simulink model "TosAeb"  (R2021a)
settings: Solver=FixedStepDiscrete  StartTime=0.0  StopTime=inf  FixedStep=0.01  RelTol=1e-3
undefined symbols (not defined in the file or in its folder): ACCModSts, AccSts1, AEB_ObjectMovingOrientation, AEB_ObjectMovingState, CN_BTCActive, CN_CurrentGearR, CN_CurrentGearR_DHT, CN_PTCActive, CN_VDCActive, CN_VSC_EspOff, CN_VSC_FCW_AEB_Error, CN_VSC_FCW_AEB_NoDispay, CN_VSC_FCW_AEB_PerfmDgdtn, CN_VSC_FCW_AEB_TempUnAvlbl, CN_VSC_VehStandstill, CNaccelAvoidMax, CNaccelAvoidMin, CNbrakePedalPress, CNcomfortStrLeftAccelMax, CNcomfortStrLeftAccelMin, CNcomfortStrRightAccelMax, CNcomfortStrRightAccelMin, CNDrvDoorOpen, CNDrvModeEco, CNDrvModeNorm, CNDrvModeOther, CNDrvModeSport, CNDrvSBRreminder, CNNull, CNobjInvalidID, CNPassDoorOpen, CNPassSBRreminder, CNPEBSAccelNegInf, CNPEBSAccelNull, CNPEBSTimeInf, CNPEBSTimeNull, CNSTOPMAX, CNSTOPNULL, CNsubjWidth, CNSwtOFF, CNSwtON, CNTTCMAX, CNTTCNULL, CNVehConfigAEBCycNone, CNVehConfigAEBNone, CNVehConfigAEBPedNone, CNVehConfigFCWNone, CNVehConfigJANone, CollisionSeverity, FALSE, FCWSnvty, JACycObjCalcInfoDefaultParams, JAPedObjCalcInfoDefaultParams, JAVehObjCalcInfoDefaultParams, latCycObjCalcInfoDefaultParams, latPedObjCalcInfoDefaultParams, longObjCalcInfoDefaultParams, ModSts, movingStatus, ObjectType ... (+1112)
libraries: simulink x3031, AEBSFunctionLib x50, Ta_Library x33, AEBSStateMachineLib x23, Mylibrary x12, simulink_extras x7, ObjSelect_lib x3, dspstat3 x2
(part 70 of 102)

== system TosAeb/FcwAebJa_Step_sys/ALG_AEBSystem/ALG_AEBController/AEB_LatObjInfoCalcApp/LatCycObjCalc/LatCycObjCalc/latCycObjInfoCalc/ObjColiisionRiskProbCalc/CollisionRiskProbCalc/CollisionRiskProbCalc/CriticalityPointCalc ==
  dxReginObjLLCorr : Inport
  dyReginObjRUCorr : Inport  Port=2
  dyReginObjLLCorr : Inport  Port=3
  Add : Sum
  Gain : Gain  Gain=0.5
  dxPreCollison : Outport
  dyPreCollison : Outport  Port=2
  wires:
  Gain/1 -> dyPreCollison/1
  Add/1 -> Gain/1
  dyReginObjLLCorr/1 -> Add/2
  dyReginObjRUCorr/1 -> Add/1
  dxReginObjLLCorr/1 -> dxPreCollison/1

== system TosAeb/FcwAebJa_Step_sys/ALG_AEBSystem/ALG_AEBController/AEB_LatObjInfoCalcApp/LatCycObjCalc/LatCycObjCalc/latCycObjInfoCalc/ObjColiisionRiskProbCalc/CollisionRiskProbCalc/CollisionRiskProbCalc/pedCollisionRiskProbCalc ==
  dyOverlap : Inport
  dyOverlapLimit : Inport  Port=2
  dxOverlap : Inport  Port=3
  dxOverlapLimit : Inport  Port=4
  Constant : Constant  OutDataTypeStr=single
  Constant1 : Constant  OutDataTypeStr=single
  Constant2 : Constant  OutDataTypeStr=single
  Divide : Product  Inputs=*/
  Divide1 : Product  Inputs=*/
  MinMax : MinMax  Inputs=2
  MinMax1 : MinMax  Inputs=2
  MinMax2 : MinMax  Inputs=2
  Product : Product
  collisionRiskProb : Outport
  dyCollisionRiskProb : Outport  Port=2
  dxCollisionRiskProb : Outport  Port=3
  wires:
  MinMax2/1 -> collisionRiskProb/1
  Constant2/1 -> MinMax2/2
  Product/1 -> MinMax2/1
  Divide1/1 -> MinMax1/1  "dxCollisionRiskProb"
  dxOverlapLimit/1 -> Divide1/2
  dxOverlap/1 -> Divide1/1
  Constant1/1 -> MinMax1/2
  MinMax1/1 -> Product/2, dxCollisionRiskProb/1  "dxCollisionRiskProb"
  dyOverlapLimit/1 -> Divide/2
  dyOverlap/1 -> Divide/1
  Divide/1 -> MinMax/1  "dyCollisionRiskProb"
  Constant/1 -> MinMax/2
  MinMax/1 -> dyCollisionRiskProb/1, Product/1  "dyCollisionRiskProb"

== system TosAeb/FcwAebJa_Step_sys/ALG_AEBSystem/ALG_AEBController/AEB_LatObjInfoCalcApp/LatCycObjCalc/LatCycObjCalc/latCycObjInfoCalc/ObjColiisionRiskProbCalc/CollisionRiskProbCalc/ObjAbsInfoCalc/RadicalRelevantCalc ==
  dyTargetObjCalc : Inport
  dxTargetObjCalc : Inport  Port=2
  subjYawRate : Inport  Port=3
  Math Function : Math  Operator=hypot  SignedPower=on  SaturateOnIntegerOverflow=on
  Product1 : Product
  Trigonometric Function : Trigonometry  Operator=atan2
  radialVelRel : Outport
  objAngleToRear : Outport  Port=2
  wires:
  dxTargetObjCalc/1 -> Math Function/1, Trigonometric Function/2
  dyTargetObjCalc/1 -> Math Function/2, Trigonometric Function/1
  Product1/1 -> radialVelRel/1  "radialVelRel"
  Math Function/1 -> Product1/1  "distRadial"
  subjYawRate/1 -> Product1/2
  Trigonometric Function/1 -> objAngleToRear/1

== system TosAeb/FcwAebJa_Step_sys/ALG_AEBSystem/ALG_AEBController/AEB_LatObjInfoCalcApp/LatCycObjCalc/LatCycObjCalc/latCycObjInfoCalc/ObjColiisionRiskProbCalc/CollisionRiskProbCalc/ObjAbsInfoCalc/RadicalToXYTrans ==
  subjVel : Inport
  velRadicalXY : Inport  Port=2
  sincosCoef : Inport  Port=3
  Add3 : Sum  Inputs=++-
  Add4 : Sum
  Bus Creator : BusCreator  Inputs=2
  Bus Creator4 : BusCreator  Inputs=2
  Bus Selector10 : BusSelector  OutputSignals=velRadicalXY.velRadicalY
  Bus Selector11 : BusSelector  OutputSignals=sincosCoef.sinCoef
  Bus Selector12 : BusSelector  OutputSignals=velRadicalXY.velRadicalX
  Bus Selector13 : BusSelector  OutputSignals=sincosCoef.sinCoef
  Bus Selector14 : BusSelector  OutputSignals=velRadicalXY.velRadicalY
  Bus Selector15 : BusSelector  OutputSignals=sincosCoef.cosCoef
  Bus Selector8 : BusSelector  OutputSignals=velRadicalXY.velRadicalX
  Bus Selector9 : BusSelector  OutputSignals=sincosCoef.cosCoef
  Product{7..10} (4 alike) : Product
  velAbsObjCorr : Outport
  wires:
  Bus Creator/1 -> velAbsObjCorr/1
  sincosCoef/1 -> Bus Creator4/2
  velRadicalXY/1 -> Bus Creator4/1
  Bus Selector12/1 -> Product9/1  "<velRadicalX>"
  Product9/1 -> Add4/1
  Bus Selector8/1 -> Product7/1  "<velRadicalX>"
  Bus Selector15/1 -> Product10/2  "<cosCoef>"
  Product10/1 -> Add4/2
  Bus Selector9/1 -> Product7/2  "<cosCoef>"
  Bus Creator4/1 -> Bus Selector8/1, Bus Selector9/1, Bus Selector10/1, Bus Selector11/1, Bus Selector12/1, Bus Selector13/1, Bus Selector14/1, Bus Selector15/1
  Add4/1 -> Bus Creator/2  "vyAbsObjCorr"
  subjVel/1 -> Add3/1
  Bus Selector10/1 -> Product8/1  "<velRadicalY>"
  Bus Selector11/1 -> Product8/2  "<sinCoef>"
  Bus Selector14/1 -> Product10/1  "<velRadicalY>"
  Product7/1 -> Add3/2
  Add3/1 -> Bus Creator/1  "vxAbsObjCorr"
  Bus Selector13/1 -> Product9/2  "<sinCoef>"
  Product8/1 -> Add3/3

== system TosAeb/FcwAebJa_Step_sys/ALG_AEBSystem/ALG_AEBController/AEB_LatObjInfoCalcApp/LatCycObjCalc/LatCycObjCalc/latCycObjInfoCalc/ObjColiisionRiskProbCalc/CollisionRiskProbCalc/ObjAbsInfoCalc/XYToRadicalTrans ==
  LongLatVelRel : Inport
  radialVelRel : Inport  Port=2
  sincosCoef : Inport  Port=3
  Add : Sum
  Add2 : Sum  Inputs=+-+
  Bus Creator2 : BusCreator  Inputs=2
  Bus Creator3 : BusCreator  Inputs=2
  Bus Selector : BusSelector  OutputSignals=LongLatVelRel.vxRelTargetObjCalc
  Bus Selector1 : BusSelector  OutputSignals=sincosCoef.cosCoef
  Bus Selector2 : BusSelector  OutputSignals=LongLatVelRel.vyRelTargetObjCalc
  Bus Selector3 : BusSelector  OutputSignals=sincosCoef.sinCoef
  Bus Selector4 : BusSelector  OutputSignals=LongLatVelRel.vyRelTargetObjCalc
  Bus Selector5 : BusSelector  OutputSignals=sincosCoef.cosCoef
  Bus Selector6 : BusSelector  OutputSignals=LongLatVelRel.vxRelTargetObjCalc
  Bus Selector7 : BusSelector  OutputSignals=sincosCoef.sinCoef
  Product{2,4..6} (4 alike) : Product
  velRadicalXY : Outport
  wires:
  LongLatVelRel/1 -> Bus Creator2/1
  sincosCoef/1 -> Bus Creator2/2
  Bus Selector7/1 -> Product6/2  "<sinCoef>"
  Bus Selector/1 -> Product2/1  "<vxRelTargetObjCalc>"
  Bus Selector3/1 -> Product4/2  "<sinCoef>"
  radialVelRel/1 -> Add2/3
  Bus Selector1/1 -> Product2/2  "<cosCoef>"
  Bus Selector4/1 -> Product5/1  "<vyRelTargetObjCalc>"
  Add/1 -> Bus Creator3/1  "velRadicalX"
  Bus Selector6/1 -> Product6/1  "<vxRelTargetObjCalc>"
  Add2/1 -> Bus Creator3/2  "velRadicalY"
  Bus Selector5/1 -> Product5/2  "<cosCoef>"
  Bus Creator2/1 -> Bus Selector/1, Bus Selector1/1, Bus Selector2/1, Bus Selector3/1, Bus Selector4/1, Bus Selector5/1, Bus Selector6/1, Bus Selector7/1
  Product2/1 -> Add/1
  Product4/1 -> Add/2
  Bus Selector2/1 -> Product4/1  "<vyRelTargetObjCalc>"
  Product5/1 -> Add2/1
  Bus Creator3/1 -> velRadicalXY/1  "velRadicalXY"
  Product6/1 -> Add2/2

== system TosAeb/FcwAebJa_Step_sys/ALG_AEBSystem/ALG_AEBController/AEB_LatObjInfoCalcApp/LatCycObjCalc/LatCycObjCalc/latCycObjInfoCalc/ObjColiisionRiskProbCalc/CollisionRiskProbCalc/ObjAbsInfoCalc/sincosCoefCalc ==
  angle : Inport
  Bus Creator : BusCreator  Inputs=2
  Sincos : Trigonometry  Operator=sincos
  sincosCoef : Outport
  wires:
  Bus Creator/1 -> sincosCoef/1  "sincosCoef"
  Sincos/2 -> Bus Creator/2  "cosCoef"
  angle/1 -> Sincos/1
  Sincos/1 -> Bus Creator/1  "sinCoef"

== system TosAeb/FcwAebJa_Step_sys/ALG_AEBSystem/ALG_AEBController/AEB_LatObjInfoCalcApp/LatCycObjCalc/LatCycObjCalc/latCycObjInfoCalc/ObjColiisionRiskProbCalc/CollisionRiskProbCalc/ObjReginCorrCalc/AccelXYMaxMinCorrCalc ==
  maxAccelDecel : Inport
  velAbsTargetCorr : Inport  Port=2
  AccelXMaxMinCorrCalc : Subsystem
  AccelXYMaxMinCalc : Subsystem
  AccelYMaxMinCorrCalc : Subsystem
  Bus Creator : BusCreator  Inputs=4
  Bus Selector : BusSelector  OutputSignals=vxAbsObjCorr,vyAbsObjCorr
  Bus Selector1 : BusSelector  OutputSignals=AccelYMax,AccelYMin
  Bus Selector2 : BusSelector  OutputSignals=AccelXMax,AccelXMin
  sincosCoefCalc : Subsystem
  vXYAngleCalc : Subsystem
  AccelXMaxMinCorr : Outport
  AccelYMaxMinCorr : Outport  Port=2
  wires:
  AccelYMaxMinCorrCalc/1(AccelYMaxMinCorr) -> AccelYMaxMinCorr/1
  AccelXMaxMinCorrCalc/1(AccelXMaxMinCorr) -> AccelXMaxMinCorr/1
  [vyAbsTargetCorr] -> AccelYMaxMinCorrCalc/1(vyAbsTargetCorr)
  Bus Selector/2 -> [vyAbsTargetCorr]  "<vyAbsObjCorr>"
  Bus Selector2/2 -> AccelXMaxMinCorrCalc/3(AccelMinX)  "<AccelXMin>"
  Bus Selector2/1 -> AccelXMaxMinCorrCalc/2(AccelMaxX)  "<AccelXMax>"
  Bus Selector1/2 -> AccelYMaxMinCorrCalc/3(AccelMinY)  "<AccelYMin>"
  Bus Selector1/1 -> AccelYMaxMinCorrCalc/2(AccelMaxY)  "<AccelYMax>"
  Bus Creator/1 -> Bus Selector1/1, Bus Selector2/1
  AccelXYMaxMinCalc/1(AccelXMax) -> Bus Creator/1  "AccelXMax"
  AccelXYMaxMinCalc/2(AccelXMin) -> Bus Creator/2  "AccelXMin"
  AccelXYMaxMinCalc/3(AccelYMax) -> Bus Creator/3  "AccelYMax"
  AccelXYMaxMinCalc/4(AccelYMin) -> Bus Creator/4  "AccelYMin"
  Bus Selector/1 -> AccelXMaxMinCorrCalc/1(vxAbsTargetCorr)  "<vxAbsObjCorr>"
  sincosCoefCalc/1(sincosCoef) -> AccelXYMaxMinCalc/2(sincosCoef)
  maxAccelDecel/1 -> AccelXYMaxMinCalc/1(maxAccelDecel)
  vXYAngleCalc/1(vXYAngle) -> sincosCoefCalc/1(angle)
  velAbsTargetCorr/1 -> vXYAngleCalc/1(velAbsTargetCorr), Bus Selector/1

== system TosAeb/FcwAebJa_Step_sys/ALG_AEBSystem/ALG_AEBController/AEB_LatObjInfoCalcApp/LatCycObjCalc/LatCycObjCalc/latCycObjInfoCalc/ObjColiisionRiskProbCalc/CollisionRiskProbCalc/ObjReginCorrCalc/CycMaxAccelDecelCalc ==
  Bus Creator : BusCreator  Inputs=2
  P_LatCycCA_CollisionRiskObjAccelMax : Constant  Value=P_AEB_LatCycCA_CollisionRiskObjAccelMax  OutDataTypeStr=single
  P_LatCycCA_CollisionRiskObjDecelMax : Constant  Value=P_AEB_LatCycCA_CollisionRiskObjDecelMax  OutDataTypeStr=single
  maxAccelDecel : Outport
  wires:
  Bus Creator/1 -> maxAccelDecel/1
  P_LatCycCA_CollisionRiskObjDecelMax/1 -> Bus Creator/2  "maxDecel"
  P_LatCycCA_CollisionRiskObjAccelMax/1 -> Bus Creator/1  "maxAccel"

== system TosAeb/FcwAebJa_Step_sys/ALG_AEBSystem/ALG_AEBController/AEB_LatObjInfoCalcApp/LatCycObjCalc/LatCycObjCalc/latCycObjInfoCalc/ObjColiisionRiskProbCalc/CollisionRiskProbCalc/ObjReginCorrCalc/ObjFrameCalc ==
  dx : Inport
  dy : Inport  Port=2
  ObjLengthCorr : Inport  Port=3
  ObjWidthCorr : Inport  Port=4
  Add : Sum
  Add1 : Sum
  Add2 : Sum  Inputs=+-
  Add3 : Sum  Inputs=+-
  Gain : Gain  Gain=0.5
  Gain1 : Gain  Gain=0.5
  Gain2 : Gain  Gain=0.5
  dxMax : Outport
  dxMin : Outport  Port=2
  dyMax : Outport  Port=3
  dyMin : Outport  Port=4
  wires:
  Add2/1 -> dyMin/1
  Add1/1 -> dyMax/1
  ObjWidthCorr/1 -> Gain/1
  dy/1 -> Add1/1, Add2/1
  Add/1 -> dxMax/1
  ObjLengthCorr/1 -> Gain2/1, Gain1/1
  Gain1/1 -> Add/2
  Gain/1 -> Add2/2, Add1/2
  dx/1 -> Add3/1, Add/1
  Add3/1 -> dxMin/1
  Gain2/1 -> Add3/2

== system TosAeb/FcwAebJa_Step_sys/ALG_AEBSystem/ALG_AEBController/AEB_LatObjInfoCalcApp/LatCycObjCalc/LatCycObjCalc/latCycObjInfoCalc/ObjColiisionRiskProbCalc/CollisionRiskProbCalc/ObjReginCorrCalc/ObjLengthWidthCalc ==
  ObjectTypeCameraConfirmed : Inport
  ObjectLength : Inport  Port=2
  ObjectWidth : Inport  Port=3
  ObjectYawAngle : Inport  Port=4
  ObjectMovingOrientation : Inport  Port=5
  ObjLengthWidthCalc : Stateflow chart  Commented=on  TreatAsAtomicUnit=on
  ObjLengthWidthCalc1 : Stateflow chart  TreatAsAtomicUnit=on
  P_LatPedCA_CollisionRiskProbLengthThres : Constant  Value=P_AEB_LatCycCA_CollisionRiskProbLengthThres
  P_LatPedCA_CollisionRiskProbWidthThres : Constant  Value=P_AEB_LatCycCA_CollisionRiskProbWidthThres
  ObjLengthCorr : Outport
  ObjWidthCorr : Outport  Port=2
  wires:
  P_LatPedCA_CollisionRiskProbLengthThres/1 -> ObjLengthWidthCalc1/6(P_LatCycCA_CollisionRiskProbLengthThres)
  P_LatPedCA_CollisionRiskProbWidthThres/1 -> ObjLengthWidthCalc1/7(P_LatCycCA_CollisionRiskProbWidthThres)
  ObjectMovingOrientation/1 -> ObjLengthWidthCalc1/5(ObjectMovingOrientation)
  ObjectYawAngle/1 -> ObjLengthWidthCalc1/4(ObjectYawAngle)
  ObjectLength/1 -> ObjLengthWidthCalc1/2(ObjectLengthIn)
  ObjectWidth/1 -> ObjLengthWidthCalc1/3(ObjectWidthIn)
  ObjectTypeCameraConfirmed/1 -> ObjLengthWidthCalc1/1(ObjectTypeCameraConfirmed)
  ObjLengthWidthCalc1/1(ObjectLength) -> ObjLengthCorr/1
  ObjLengthWidthCalc1/2(ObjectWidth) -> ObjWidthCorr/1

== system TosAeb/FcwAebJa_Step_sys/ALG_AEBSystem/ALG_AEBController/AEB_LatObjInfoCalcApp/LatCycObjCalc/LatCycObjCalc/latCycObjInfoCalc/ObjColiisionRiskProbCalc/CollisionRiskProbCalc/ObjReginCorrCalc/ObjLengthWidthCalc1 ==
  ObjectTypeCameraConfirmed : Inport
  ObjectLength : Inport  Port=2
  ObjectWidth : Inport  Port=3
  ObjectYawAngle : Inport  Port=4
  ObjectMovingOrientation : Inport  Port=5
  ObjLengthWidthCalc : Stateflow chart  Commented=on  TreatAsAtomicUnit=on
  ObjLengthWidthCalc1 : Stateflow chart  TreatAsAtomicUnit=on
  P_LatPedCA_CollisionRiskProbLengthThres : Constant  Value=P_AEB_LatPedCA_CollisionRiskProbLengthThres
  P_LatPedCA_CollisionRiskProbWidthThres : Constant  Value=P_AEB_LatPedCA_CollisionRiskProbWidthThres
  ObjLengthCorr : Outport
  ObjWidthCorr : Outport  Port=2
  wires:
  P_LatPedCA_CollisionRiskProbLengthThres/1 -> ObjLengthWidthCalc1/6(P_LatPedCA_CollisionRiskProbLengthThres)
  ObjectTypeCameraConfirmed/1 -> ObjLengthWidthCalc1/1(ObjectTypeCameraConfirmed)
  ObjectLength/1 -> ObjLengthWidthCalc1/2(ObjectLengthIn)
  ObjectWidth/1 -> ObjLengthWidthCalc1/3(ObjectWidthIn)
  ObjectYawAngle/1 -> ObjLengthWidthCalc1/4(ObjectYawAngle)
  ObjectMovingOrientation/1 -> ObjLengthWidthCalc1/5(ObjectMovingOrientation)
  P_LatPedCA_CollisionRiskProbWidthThres/1 -> ObjLengthWidthCalc1/7(P_LatPedCA_CollisionRiskProbWidthThres)
  ObjLengthWidthCalc1/2(ObjectWidth) -> ObjWidthCorr/1
  ObjLengthWidthCalc1/1(ObjectLength) -> ObjLengthCorr/1

== system TosAeb/FcwAebJa_Step_sys/ALG_AEBSystem/ALG_AEBController/AEB_LatObjInfoCalcApp/LatCycObjCalc/LatCycObjCalc/latCycObjInfoCalc/ObjColiisionRiskProbCalc/CollisionRiskProbCalc/ObjReginCorrCalc/ObjReginCorrCalc ==
  AccelXMaxMinCorr : Inport
  AccelYMaxMinCorr : Inport  Port=2
  velAbsTargetCorr : Inport  Port=3
  dxMax : Inport  Port=4
  dxMin : Inport  Port=5
  dyMax : Inport  Port=6
  dyMin : Inport  Port=7
  tPredTime : Inport  Port=8
  vxAbsObj : Inport  Port=9
  vy : Inport  Port=10
  Add{,1..3} (4 alike) : Sum  Inputs=+++
  Bus Creator : BusCreator  Inputs=7
  Bus Creator1 : BusCreator  Inputs=4
  Bus Creator2 : BusCreator  Inputs=2
  Bus Selector : BusSelector  OutputSignals=BusSig1.dxMax,BusSig1.velAbsTargetCorr.vxAbsObjCorr,tPredTime.tPredTimeMax_X,BusSig1.AccelXMaxMinCorr.AccelXMaxCorr
  Bus Selector1 : BusSelector  OutputSignals=BusSig1.dyMin,BusSig1.velAbsTargetCorr.vyAbsObjCorr,tPredTime.tPredTimeMin_Y,BusSig1.AccelYMaxMinCorr.AccelYMinCorr
  Bus Selector2 : BusSelector  OutputSignals=BusSig1.dxMin,BusSig1.velAbsTargetCorr.vxAbsObjCorr,tPredTime.tPredTimeMin_X,BusSig1.AccelXMaxMinCorr.AccelXMinCorr
  Bus Selector3 : BusSelector  OutputSignals=BusSig1.dyMax,BusSig1.velAbsTargetCorr.vyAbsObjCorr,tPredTime.tPredTimeMax_Y,BusSig1.AccelYMaxMinCorr.AccelYMaxCorr
  Bus Selector4 : BusSelector  OutputSignals=AccelXMaxMinCorr.AccelXMaxCorr,AccelXMaxMinCorr.AccelXMinCorr,AccelYMaxMinCorr.AccelYMaxCorr,AccelYMaxMinCorr.AccelYMinCorr
  Chart : Stateflow chart  TreatAsAtomicUnit=on
  Gain{,1..3} (4 alike) : Gain  Gain=0.5
  Math Function{,1..3} (4 alike) : Math  Operator=square  SignedPower=on  SaturateOnIntegerOverflow=on
  Product{,1..7} (8 alike) : Product
  dxReginObjRUCorr : Outport
  dyReginObjRUCorr : Outport  Port=2
  dxReginObjLLCorr : Outport  Port=3
  dyReginObjLLCorr : Outport  Port=4
  wires:
  Gain3/1 -> Add3/3
  Gain2/1 -> Add2/3
  Gain1/1 -> Add1/3
  Gain/1 -> Add/3
  Bus Selector3/1 -> Add3/1  "<dyMax>"
  Bus Selector3/2 -> Product6/1  "<vyAbsObjCorr>"
  Bus Selector3/3 -> Math Function3/1, Product6/2  "<tPredTimeMax_Y>"
  Product6/1 -> Add3/2
  Math Function3/1 -> Product7/1
  Bus Selector3/4 -> Product7/2  "<AccelYMaxCorr>"
  Product7/1 -> Gain3/1
  Add3/1 -> dyReginObjLLCorr/1
  Add2/1 -> dxReginObjLLCorr/1
  Product5/1 -> Gain2/1
  Bus Selector2/4 -> Product5/2  "<AccelXMinCorr>"
  Math Function2/1 -> Product5/1
  Product4/1 -> Add2/2
  Bus Selector2/3 -> Product4/2, Math Function2/1  "<tPredTimeMin_X>"
  Bus Selector2/2 -> Product4/1  "<vxAbsObjCorr>"
  Bus Selector2/1 -> Add2/1  "<dxMin>"
  Bus Selector1/1 -> Add1/1  "<dyMin>"
  Bus Selector1/2 -> Product2/1  "<vyAbsObjCorr>"
  Bus Selector1/3 -> Math Function1/1, Product2/2  "<tPredTimeMin_Y>"
  Product2/1 -> Add1/2
  Math Function1/1 -> Product3/1
  Bus Selector1/4 -> Product3/2  "<AccelYMinCorr>"
  Product3/1 -> Gain1/1
  Add1/1 -> dyReginObjRUCorr/1
  Add/1 -> dxReginObjRUCorr/1
  Product1/1 -> Gain/1
  Bus Selector/4 -> Product1/2  "<AccelXMaxCorr>"
  Math Function/1 -> Product1/1
  Product/1 -> Add/2
  Bus Selector/3 -> Product/2, Math Function/1  "<tPredTimeMax_X>"
  Bus Selector/2 -> Product/1  "<vxAbsObjCorr>"
  Bus Selector/1 -> Add/1  "<dxMax>"
  AccelXMaxMinCorr/1 -> Bus Creator/1  "AccelXMaxMinCorr"
  AccelYMaxMinCorr/1 -> Bus Creator/2  "AccelYMaxMinCorr"
  dyMin/1 -> Bus Creator/7  "dyMin"
  velAbsTargetCorr/1 -> Bus Creator/3  "velAbsTargetCorr"
  dyMax/1 -> Bus Creator/6  "dyMax"
  dxMax/1 -> Bus Creator/4  "dxMax"
  dxMin/1 -> Bus Creator/5  "dxMin"
  vxAbsObj/1 -> Chart/6(vxAbsObj)
  vy/1 -> Chart/7(vyAbsObj)
  tPredTime/1 -> Chart/5(tPredTime)
  Bus Selector4/2 -> Chart/2(ax_Min)  "<AccelXMinCorr>"
  Bus Selector4/1 -> Chart/1(ax_Max)  "<AccelXMaxCorr>"
  Bus Selector4/3 -> Chart/3(ay_Max)  "<AccelYMaxCorr>"
  Bus Selector4/4 -> Chart/4(ay_Min)  "<AccelYMinCorr>"
  Chart/1(tPredTimeMax_X) -> Bus Creator1/1  "tPredTimeMax_X"
  Chart/2(tPredTimeMin_X) -> Bus Creator1/2  "tPredTimeMin_X"
  Chart/3(tPredTimeMax_Y) -> Bus Creator1/3  "tPredTimeMax_Y"
  Chart/4(tPredTimeMin_Y) -> Bus Creator1/4  "tPredTimeMin_Y"
  Bus Creator1/1 -> Bus Creator2/2  "tPredTime"
  Bus Creator/1 -> Bus Selector4/1, Bus Creator2/1  "BusSig1"
  Bus Creator2/1 -> Bus Selector3/1, Bus Selector2/1, Bus Selector1/1, Bus Selector/1

== system TosAeb/FcwAebJa_Step_sys/ALG_AEBSystem/ALG_AEBController/AEB_LatObjInfoCalcApp/LatCycObjCalc/LatCycObjCalc/latCycObjInfoCalc/ObjCrossFlgCal/CycPreCollisionCalcFunc/ObjBoundingBox/ObjLLCoordinate ==
  inputSignals : Inport
  Add : Sum  Inputs=+-  OutDataTypeStr=single
  Add1 : Sum  OutDataTypeStr=single
  Bus Selector : BusSelector  OutputSignals=dxPreRefPointObj,ObjWidthCorr,sincosCoef.sinCoef
  Bus Selector1 : BusSelector  OutputSignals=dyPreRefPointObj,ObjWidthCorr,sincosCoef.cosCoef
  Gain : Gain  Gain=0.5  OutDataTypeStr=single
  Gain1 : Gain  Gain=0.5  OutDataTypeStr=single
  Product1 : Product  OutDataTypeStr=single
  Product3 : Product  OutDataTypeStr=single
  dxPre_LLObj : Outport
  dyPreLLObj : Outport  Port=2
  wires:
  Gain1/1 -> Add/2
  Gain/1 -> Add1/2
  inputSignals/1 -> Bus Selector/1, Bus Selector1/1
  Bus Selector/2 -> Product1/1  "<ObjWidthCorr>"
  Bus Selector/3 -> Product1/2  "<sinCoef>"
  Product1/1 -> Gain1/1
  Bus Selector/1 -> Add/1  "<dxPreRefPointObj>"
  Add/1 -> dxPre_LLObj/1
  Add1/1 -> dyPreLLObj/1
  Product3/1 -> Gain/1
  Bus Selector1/2 -> Product3/1  "<ObjWidthCorr>"
  Bus Selector1/3 -> Product3/2  "<cosCoef>"
  Bus Selector1/1 -> Add1/1  "<dyPreRefPointObj>"

== system TosAeb/FcwAebJa_Step_sys/ALG_AEBSystem/ALG_AEBController/AEB_LatObjInfoCalcApp/LatCycObjCalc/LatCycObjCalc/latCycObjInfoCalc/ObjCrossFlgCal/CycPreCollisionCalcFunc/ObjBoundingBox/ObjLUCoordinate ==
  inputSignals : Inport
  Add : Sum  Inputs=++-  OutDataTypeStr=single
  Add1 : Sum  Inputs=+++  OutDataTypeStr=single
  Bus Selector : BusSelector  OutputSignals=dxPreRefPointObj,ObjLengthCorr,sincosCoef.cosCoef,ObjWidthCorr,sincosCoef.sinCoef
  Bus Selector1 : BusSelector  OutputSignals=dyPreRefPointObj,ObjLengthCorr,sincosCoef.sinCoef,ObjWidthCorr,sincosCoef.cosCoef
  Gain : Gain  Gain=0.5  OutDataTypeStr=single
  Gain1 : Gain  Gain=0.5  OutDataTypeStr=single
  Product{,1..3} (4 alike) : Product  OutDataTypeStr=single
  dxPreLUObj : Outport
  dyPreLUObj : Outport  Port=2
  wires:
  Gain1/1 -> Add/3
  Gain/1 -> Add1/3
  Bus Selector1/1 -> Add1/1  "<dyPreRefPointObj>"
  Bus Selector1/5 -> Product3/2  "<cosCoef>"
  Bus Selector1/3 -> Product2/2  "<sinCoef>"
  Bus Selector1/4 -> Product3/1  "<ObjWidthCorr>"
  Bus Selector1/2 -> Product2/1  "<ObjLengthCorr>"
  Product3/1 -> Gain/1
  Product2/1 -> Add1/2
  Add1/1 -> dyPreLUObj/1
  Add/1 -> dxPreLUObj/1
  Product/1 -> Add/2
  Bus Selector/1 -> Add/1  "<dxPreRefPointObj>"
  Product1/1 -> Gain1/1
  Bus Selector/5 -> Product1/2  "<sinCoef>"
  Bus Selector/4 -> Product1/1  "<ObjWidthCorr>"
  Bus Selector/3 -> Product/2  "<cosCoef>"
  Bus Selector/2 -> Product/1  "<ObjLengthCorr>"
  inputSignals/1 -> Bus Selector/1, Bus Selector1/1

== system TosAeb/FcwAebJa_Step_sys/ALG_AEBSystem/ALG_AEBController/AEB_LatObjInfoCalcApp/LatCycObjCalc/LatCycObjCalc/latCycObjInfoCalc/ObjCrossFlgCal/CycPreCollisionCalcFunc/ObjBoundingBox/ObjRLCoordinate ==
  inputSignals : Inport
  Add : Sum  OutDataTypeStr=single
  Add1 : Sum  Inputs=+-  OutDataTypeStr=single
  Bus Selector : BusSelector  OutputSignals=dxPreRefPointObj,ObjWidthCorr,sincosCoef.sinCoef
  Bus Selector1 : BusSelector  OutputSignals=dyPreRefPointObj,ObjWidthCorr,sincosCoef.cosCoef
  Gain : Gain  Gain=0.5  OutDataTypeStr=single
  Gain1 : Gain  Gain=0.5  OutDataTypeStr=single
  Product1 : Product  OutDataTypeStr=single
  Product3 : Product  OutDataTypeStr=single
  dxPreRLObj : Outport
  dyPreRLObj : Outport  Port=2
  wires:
  Gain1/1 -> Add1/2
  Gain/1 -> Add/2
  inputSignals/1 -> Bus Selector/1, Bus Selector1/1
  Bus Selector/2 -> Product1/1  "<ObjWidthCorr>"
  Bus Selector/3 -> Product1/2  "<sinCoef>"
  Product1/1 -> Gain/1
  Bus Selector/1 -> Add/1  "<dxPreRefPointObj>"
  Add/1 -> dxPreRLObj/1
  Add1/1 -> dyPreRLObj/1
  Product3/1 -> Gain1/1
  Bus Selector1/2 -> Product3/1  "<ObjWidthCorr>"
  Bus Selector1/3 -> Product3/2  "<cosCoef>"
  Bus Selector1/1 -> Add1/1  "<dyPreRefPointObj>"

== system TosAeb/FcwAebJa_Step_sys/ALG_AEBSystem/ALG_AEBController/AEB_LatObjInfoCalcApp/LatCycObjCalc/LatCycObjCalc/latCycObjInfoCalc/ObjCrossFlgCal/CycPreCollisionCalcFunc/ObjBoundingBox/ObjRUCoordinate ==
  inputSignals : Inport
  Add : Sum  Inputs=+++  OutDataTypeStr=single
  Add1 : Sum  Inputs=++-  OutDataTypeStr=single
  Bus Selector : BusSelector  OutputSignals=dxPreRefPointObj,ObjLengthCorr,sincosCoef.cosCoef,ObjWidthCorr,sincosCoef.sinCoef
  Bus Selector1 : BusSelector  OutputSignals=dyPreRefPointObj,ObjLengthCorr,sincosCoef.sinCoef,ObjWidthCorr,sincosCoef.cosCoef
  Gain : Gain  Gain=0.5  OutDataTypeStr=single
  Gain1 : Gain  Gain=0.5  OutDataTypeStr=single
  Product{,1..3} (4 alike) : Product  OutDataTypeStr=single
  dxPreRUObj : Outport
  dyPreRUObj : Outport  Port=2
  wires:
  Gain1/1 -> Add/3
  Gain/1 -> Add1/3
  Bus Selector1/1 -> Add1/1  "<dyPreRefPointObj>"
  Bus Selector1/5 -> Product3/2  "<cosCoef>"
  Bus Selector1/3 -> Product2/2  "<sinCoef>"
  Bus Selector1/4 -> Product3/1  "<ObjWidthCorr>"
  Bus Selector1/2 -> Product2/1  "<ObjLengthCorr>"
  Product3/1 -> Gain/1
  Product2/1 -> Add1/2
  Add1/1 -> dyPreRUObj/1
  Add/1 -> dxPreRUObj/1
  Product/1 -> Add/2
  Bus Selector/1 -> Add/1  "<dxPreRefPointObj>"
  Product1/1 -> Gain1/1
  Bus Selector/5 -> Product1/2  "<sinCoef>"
  Bus Selector/4 -> Product1/1  "<ObjWidthCorr>"
  Bus Selector/3 -> Product/2  "<cosCoef>"
  Bus Selector/2 -> Product/1  "<ObjLengthCorr>"
  inputSignals/1 -> Bus Selector/1, Bus Selector1/1

== system TosAeb/FcwAebJa_Step_sys/ALG_AEBSystem/ALG_AEBController/AEB_LatObjInfoCalcApp/LatCycObjCalc/LatCycObjCalc/latCycObjInfoCalc/ObjCrossFlgCal/CycPreCollisionCalcFunc/PreCrossFlgCalcFlow/VectorCrossAlgorithm/If Action Subsystem ==
  Action Port : ActionPort  ActionPortLabel=if(u1)
  Constant : Constant  Value=0  OutDataTypeStr=boolean
  CrossFlg : Outport
  wires:
  Constant/1 -> CrossFlg/1

== system TosAeb/FcwAebJa_Step_sys/ALG_AEBSystem/ALG_AEBController/AEB_LatObjInfoCalcApp/LatCycObjCalc/LatCycObjCalc/latCycObjInfoCalc/ObjCrossFlgCal/CycPreCollisionCalcFunc/PreCrossFlgCalcFlow/VectorCrossAlgorithm/If Action Subsystem1 ==
  vectorMulit : Inport
  Coordinations : Inport  Port=2
  Action Port : ActionPort  ActionPortLabel=else
  Bus Selector : BusSelector  OutputSignals=m2,m1,y1,n1,n2,x1
  Bus Selector1 : BusSelector  OutputSignals=x2,x1,y1,n1,y2,m1
  Subsystem : Subsystem
  t : Subsystem
  u : Subsystem
  CrossFlg : Outport
  wires:
  vectorMulit/1 -> Subsystem/3(vectorMulit)
  Subsystem/1(CrossFlg) -> CrossFlg/1
  u/1(temp_u) -> Subsystem/2(temp_t)
  t/1(temp_t) -> Subsystem/1(temp_u)
  Bus Selector1/6 -> u/6(m1)  "<m1>"
  Bus Selector1/5 -> u/5(y2)  "<y2>"
  Bus Selector1/1 -> u/1(x2)  "<x2>"
  Bus Selector1/4 -> u/4(n1)  "<n1>"
  Bus Selector1/2 -> u/2(x1)  "<x1>"
  Bus Selector1/3 -> u/3(y1)  "<y1>"
  Bus Selector/6 -> t/6(x1)  "<x1>"
  Bus Selector/5 -> t/5(n2)  "<n2>"
  Bus Selector/4 -> t/4(n1)  "<n1>"
  Bus Selector/1 -> t/1(m2)  "<m2>"
  Bus Selector/3 -> t/3(y1)  "<y1>"
  Bus Selector/2 -> t/2(m1)  "<m1>"
  Coordinations/1 -> Bus Selector/1, Bus Selector1/1

== system TosAeb/FcwAebJa_Step_sys/ALG_AEBSystem/ALG_AEBController/AEB_LatObjInfoCalcApp/LatCycObjCalc/LatCycObjCalc/latCycObjInfoCalc/ObjCrossFlgCal/CycPreCollisionCalcFunc/PreCrossFlgCalcFlow/VectorCrossAlgorithm/VectorMulit ==
  x2 : Inport  OutDataTypeStr=single
  x1 : Inport  Port=2  OutDataTypeStr=single
  n2 : Inport  Port=3  OutDataTypeStr=single
  n1 : Inport  Port=4  OutDataTypeStr=single
  y2 : Inport  Port=5  OutDataTypeStr=single
  y1 : Inport  Port=6  OutDataTypeStr=single
  m2 : Inport  Port=7  OutDataTypeStr=single
  m1 : Inport  Port=8  OutDataTypeStr=single
  Product : Product  OutDataTypeStr=single
  Product1 : Product  OutDataTypeStr=single
  Subtract{1..4} (4 alike) : Sum  Inputs=+-  OutDataTypeStr=single
  Subtract5 : Sum  Inputs=+-
  vectorMulit : Outport
  wires:
  x1/1 -> Subtract2/2
  x2/1 -> Subtract2/1
  n1/1 -> Subtract3/2
  n2/1 -> Subtract3/1
  Subtract5/1 -> vectorMulit/1
  Product1/1 -> Subtract5/2
  Product/1 -> Subtract5/1
  Subtract1/1 -> Product1/2
  Subtract4/1 -> Product1/1
  m1/1 -> Subtract1/2
  m2/1 -> Subtract1/1
  y1/1 -> Subtract4/2
  y2/1 -> Subtract4/1
  Subtract3/1 -> Product/2
  Subtract2/1 -> Product/1

== system TosAeb/FcwAebJa_Step_sys/ALG_AEBSystem/ALG_AEBController/AEB_LatObjInfoCalcApp/LatCycObjCalc/LatCycObjCalc/latCycObjInfoCalc/ObjCrossFlgCal/CycPreCollisionCalcFunc/SubjBoundingBox/SubjLLCoordinate ==
  inputSignals : Inport
  Add2 : Sum  Inputs=+--  OutDataTypeStr=single
  Add3 : Sum  Inputs=+-+  OutDataTypeStr=single
  Bus Selector2 : BusSelector  OutputSignals=dxPreRefPoint,dxRearBumperToRearAxis,sincosCoef.cosCoef,subjWidth,sincosCoef.sinCoef
  Bus Selector3 : BusSelector  OutputSignals=dyPreRefPoint,dxRearBumperToRearAxis,sincosCoef.sinCoef,subjWidth,sincosCoef.cosCoef
  Gain2 : Gain  Gain=0.5  OutDataTypeStr=single
  Gain3 : Gain  Gain=0.5  OutDataTypeStr=single
  Product{4..7} (4 alike) : Product  OutDataTypeStr=single
  dxPreLL : Outport
  dyPreLL : Outport  Port=2
  wires:
  Bus Selector2/5 -> Product5/2  "<sinCoef>"
  Bus Selector2/1 -> Add2/1  "<dxPreRefPoint>"
  Bus Selector2/3 -> Product4/2  "<cosCoef>"
  Bus Selector2/2 -> Product4/1  "<dxRearBumperToRearAxis>"
  Bus Selector2/4 -> Product5/1  "<subjWidth>"
  inputSignals/1 -> Bus Selector2/1, Bus Selector3/1
  Bus Selector3/1 -> Add3/1  "<dyPreRefPoint>"
  Bus Selector3/2 -> Product6/1  "<dxRearBumperToRearAxis>"
  Bus Selector3/4 -> Product7/1  "<subjWidth>"
  Add2/1 -> dxPreLL/1
  Gain2/1 -> Add2/3
  Product5/1 -> Gain2/1
  Bus Selector3/5 -> Product7/2  "<cosCoef>"
  Product4/1 -> Add2/2
  Product6/1 -> Add3/2
  Product7/1 -> Gain3/1
  Add3/1 -> dyPreLL/1
  Bus Selector3/3 -> Product6/2  "<sinCoef>"
  Gain3/1 -> Add3/3

== system TosAeb/FcwAebJa_Step_sys/ALG_AEBSystem/ALG_AEBController/AEB_LatObjInfoCalcApp/LatCycObjCalc/LatCycObjCalc/latCycObjInfoCalc/ObjCrossFlgCal/CycPreCollisionCalcFunc/SubjBoundingBox/SubjLUCoordinate ==
  inputSignals : Inport
  Add : Sum  Inputs=++-  OutDataTypeStr=single
  Add1 : Sum  Inputs=+++  OutDataTypeStr=single
  Bus Selector : BusSelector  OutputSignals=dxPreRefPoint,dxFrontBumperToRearAxis,sincosCoef.cosCoef,subjWidth,sincosCoef.sinCoef
  Bus Selector1 : BusSelector  OutputSignals=dyPreRefPoint,dxFrontBumperToRearAxis,sincosCoef.sinCoef,subjWidth,sincosCoef.cosCoef
  Gain : Gain  Gain=0.5  OutDataTypeStr=single
  Gain1 : Gain  Gain=0.5  OutDataTypeStr=single
  Product{,1..3} (4 alike) : Product  OutDataTypeStr=single
  dxPreLU : Outport
  dyPreLU : Outport  Port=2
  wires:
  Bus Selector1/3 -> Product2/2  "<sinCoef>"
  Product/1 -> Add/2
  Product1/1 -> Gain/1
  Gain/1 -> Add/3
  Bus Selector/1 -> Add/1  "<dxPreRefPoint>"
  Bus Selector1/5 -> Product3/2  "<cosCoef>"
  inputSignals/1 -> Bus Selector/1, Bus Selector1/1
  Bus Selector1/2 -> Product2/1  "<dxFrontBumperToRearAxis>"
  Bus Selector/2 -> Product/1  "<dxFrontBumperToRearAxis>"
  Bus Selector/5 -> Product1/2  "<sinCoef>"
  Add1/1 -> dyPreLU/1
  Bus Selector/3 -> Product/2  "<cosCoef>"
  Product2/1 -> Add1/2
  Bus Selector1/4 -> Product3/1  "<subjWidth>"
  Gain1/1 -> Add1/3
  Bus Selector1/1 -> Add1/1  "<dyPreRefPoint>"
  Add/1 -> dxPreLU/1
  Product3/1 -> Gain1/1
  Bus Selector/4 -> Product1/1  "<subjWidth>"

== system TosAeb/FcwAebJa_Step_sys/ALG_AEBSystem/ALG_AEBController/AEB_LatObjInfoCalcApp/LatCycObjCalc/LatCycObjCalc/latCycObjInfoCalc/ObjCrossFlgCal/CycPreCollisionCalcFunc/SubjBoundingBox/SubjRLCoordinate ==
  inputSignals : Inport
  Add6 : Sum  Inputs=+-+  OutDataTypeStr=single
  Add7 : Sum  Inputs=+--  OutDataTypeStr=single
  Bus Selector6 : BusSelector  OutputSignals=dxPreRefPoint,dxRearBumperToRearAxis,sincosCoef.cosCoef,subjWidth,sincosCoef.sinCoef
  Bus Selector7 : BusSelector  OutputSignals=dyPreRefPoint,dxRearBumperToRearAxis,sincosCoef.sinCoef,subjWidth,sincosCoef.cosCoef
  Gain6 : Gain  Gain=0.5  OutDataTypeStr=single
  Gain7 : Gain  Gain=0.5  OutDataTypeStr=single
  Product{12..15} (4 alike) : Product  OutDataTypeStr=single
  dxPreRL : Outport
  dyPreRL : Outport  Port=2
  wires:
  Bus Selector7/3 -> Product14/2  "<sinCoef>"
  Bus Selector7/5 -> Product15/2  "<cosCoef>"
  Product15/1 -> Gain7/1
  Bus Selector7/4 -> Product15/1  "<subjWidth>"
  Bus Selector7/2 -> Product14/1  "<dxRearBumperToRearAxis>"
  Bus Selector7/1 -> Add7/1  "<dyPreRefPoint>"
  Gain7/1 -> Add7/3
  Product14/1 -> Add7/2
  Add7/1 -> dyPreRL/1
  inputSignals/1 -> Bus Selector6/1, Bus Selector7/1
  Bus Selector6/3 -> Product12/2  "<cosCoef>"
  Bus Selector6/2 -> Product12/1  "<dxRearBumperToRearAxis>"
  Bus Selector6/1 -> Add6/1  "<dxPreRefPoint>"
  Bus Selector6/5 -> Product13/2  "<sinCoef>"
  Bus Selector6/4 -> Product13/1  "<subjWidth>"
  Add6/1 -> dxPreRL/1
  Product13/1 -> Gain6/1
  Gain6/1 -> Add6/3
  Product12/1 -> Add6/2

== system TosAeb/FcwAebJa_Step_sys/ALG_AEBSystem/ALG_AEBController/AEB_LatObjInfoCalcApp/LatCycObjCalc/LatCycObjCalc/latCycObjInfoCalc/ObjCrossFlgCal/CycPreCollisionCalcFunc/SubjBoundingBox/SubjRUCoordinate ==
  inputSignals : Inport
  Add4 : Sum  Inputs=+++  OutDataTypeStr=single
  Add5 : Sum  Inputs=++-  OutDataTypeStr=single
  Bus Selector4 : BusSelector  OutputSignals=dxPreRefPoint,dxFrontBumperToRearAxis,sincosCoef.cosCoef,subjWidth,sincosCoef.sinCoef
  Bus Selector5 : BusSelector  OutputSignals=dyPreRefPoint,dxFrontBumperToRearAxis,sincosCoef.sinCoef,subjWidth,sincosCoef.cosCoef
  Gain4 : Gain  Gain=0.5  OutDataTypeStr=single
  Gain5 : Gain  Gain=0.5  OutDataTypeStr=single
  Product{8..11} (4 alike) : Product  OutDataTypeStr=single
  dxPreRU : Outport
  dyPreRU : Outport  Port=2
  wires:
  Bus Selector5/1 -> Add5/1  "<dyPreRefPoint>"
  Product8/1 -> Add4/2
  Gain4/1 -> Add4/3
  Add4/1 -> dxPreRU/1
  Bus Selector5/5 -> Product11/2  "<cosCoef>"
  Bus Selector5/3 -> Product10/2  "<sinCoef>"
  Bus Selector5/2 -> Product10/1  "<dxFrontBumperToRearAxis>"
  Gain5/1 -> Add5/3
  Add5/1 -> dyPreRU/1
  Product11/1 -> Gain5/1
  Product10/1 -> Add5/2
  Bus Selector5/4 -> Product11/1  "<subjWidth>"
  Product9/1 -> Gain4/1
  Bus Selector4/2 -> Product8/1  "<dxFrontBumperToRearAxis>"
  inputSignals/1 -> Bus Selector4/1, Bus Selector5/1
  Bus Selector4/1 -> Add4/1  "<dxPreRefPoint>"
  Bus Selector4/3 -> Product8/2  "<cosCoef>"
  Bus Selector4/5 -> Product9/2  "<sinCoef>"
  Bus Selector4/4 -> Product9/1  "<subjWidth>"

== system TosAeb/FcwAebJa_Step_sys/ALG_AEBSystem/ALG_AEBController/AEB_LatObjInfoCalcApp/LatPedObjCalc/LatPedCollisonAssessInfo/LatPedCollisonAssessInfo/LatPedLongDistCondCalc/PedDistBrakeFullCalc/DistPhase2Calc/distPhase2Calc ==
  tPhase2 : Inport  OutDataTypeStr=single
  subjAccelFilt : Inport  Port=2  OutDataTypeStr=single
  Kjerk : Inport  Port=3  OutDataTypeStr=single
  subjVel : Inport  Port=4
  Add : Sum  Inputs=3  OutDataTypeStr=single
  Constant : Constant  Value=1/6  OutDataTypeStr=single
  Constant1 : Constant  Value=0.5  OutDataTypeStr=single
  Product : Product  Inputs=5  OutDataTypeStr=single
  Product1 : Product  Inputs=4  OutDataTypeStr=single
  Product2 : Product  OutDataTypeStr=single
  distPhase2 : Outport
  wires:
  tPhase2/1 -> Product1/3, Product1/4, Product2/2, Product/1, Product/2, Product/3
  Kjerk/1 -> Product/4
  Constant/1 -> Product/5
  subjAccelFilt/1 -> Product1/2
  Constant1/1 -> Product1/1
  subjVel/1 -> Product2/1
  Product2/1 -> Add/2
  Product/1 -> Add/3
  Product1/1 -> Add/1
  Add/1 -> distPhase2/1

== system TosAeb/FcwAebJa_Step_sys/ALG_AEBSystem/ALG_AEBController/AEB_LatObjInfoCalcApp/LatPedObjCalc/LatPedCollisonAssessInfo/LatPedCollisonAssessInfo/LatPedLongDistCondCalc/PedDistBrakeFullCalc/DistPhase2Calc/tPhase2Calc ==
  note: t jerk_amax = Max[0 ，（ a m ax - a ego ） /K jerk ] ；
  note: t jerk_0 = （ -a ego /K jer k ） + sqrt （（ a ego /K jer k ） ²- 2* （ v ego / K jer k ））
  subjVel : Inport
  Kjerk : Inport  Port=2  OutDataTypeStr=single
  subjLongAccelFilt : Inport  Port=3  OutDataTypeStr=single
  accelMax : Inport  Port=4  OutDataTypeStr=single
  Add : Sum  Inputs=+-  OutDataTypeStr=single
  Add1 : Sum  Inputs=-+  OutDataTypeStr=single
  Add2 : Sum  Inputs=+-  OutDataTypeStr=single
  Constant : Constant  Value=0  OutDataTypeStr=single
  Divide : Product  Inputs=*/  OutDataTypeStr=single
  Divide1 : Product  Inputs=*/  OutDataTypeStr=single
  Divide2 : Product  Inputs=*/  OutDataTypeStr=single
  Gain : Gain  Gain=2  OutDataTypeStr=single
  Math Function : Math  Operator=square  SignedPower=on  OutDataTypeStr=single  SaturateOnIntegerOverflow=on
  MinMax : MinMax  Function=max  Inputs=2  OutDataTypeStr=single
  MinMax1 : MinMax  Inputs=2  OutDataTypeStr=single
  Relational Operator : RelationalOperator  Operator=<=  OutDataTypeStr=boolean  RndMeth=Simplest
  Sqrt : Sqrt  OutDataTypeStr=single  SaturateOnIntegerOverflow=on
  egoStopFlg : Outport
  tJerkWork : Outport  Port=2
  tPhase2 : Outport  Port=3
  wires:
  Add2/1 -> Divide2/1
  MinMax/1 -> tJerkWork/1, Relational Operator/2, MinMax1/1  "tJerkWork"
  subjLongAccelFilt/1 -> Add2/2, Divide/1
  Sqrt/1 -> Add1/2
  Kjerk/1 -> Divide2/2, Divide/2, Divide1/2
  accelMax/1 -> Add2/1
  Divide2/1 -> MinMax/1
  Constant/1 -> MinMax/2
  Divide/1 -> Add1/1, Math Function/1
  MinMax1/1 -> tPhase2/1
  Divide1/1 -> Gain/1
  Add/1 -> Sqrt/1
  Gain/1 -> Add/2
  Add1/1 -> Relational Operator/1, MinMax1/2  "tEgoStop"
  Math Function/1 -> Add/1
  subjVel/1 -> Divide1/1
  Relational Operator/1 -> egoStopFlg/1

== system TosAeb/FcwAebJa_Step_sys/ALG_AEBSystem/ALG_AEBController/AEB_LatObjInfoCalcApp/LatPedObjCalc/LatPedCollisonAssessInfo/LatPedCollisonAssessInfo/LatPedLongDistCondCalc/PedDistBrakeFullCalc/DistPhase3Calc/distPhase3Calc ==
  note: d3=（vego+ 0.5* aego*（t1 + t2）+ 0.5* Kjerk* t2²）*t3 + 0.5* amax* t3²；
  tPhase3 : Inport  OutDataTypeStr=single
  subjVel : Inport  Port=2
  subjLongAccelFilt : Inport  Port=3  OutDataTypeStr=single
  Kjerk : Inport  Port=4  OutDataTypeStr=single
  tPhase1 : Inport  Port=5  OutDataTypeStr=single
  tPhase2 : Inport  Port=6  OutDataTypeStr=single
  accelMax : Inport  Port=7  OutDataTypeStr=single
  Add : Sum  OutDataTypeStr=single
  Add1 : Sum  Inputs=+++  OutDataTypeStr=single
  Add2 : Sum  OutDataTypeStr=single
  Constant : Constant  Value=0.5  OutDataTypeStr=single
  Constant1 : Constant  Value=0.5  OutDataTypeStr=single
  Constant2 : Constant  Value=0.5  OutDataTypeStr=single
  Product : Product  Inputs=3  OutDataTypeStr=single
  Product1 : Product  Inputs=4  OutDataTypeStr=single
  Product2 : Product  OutDataTypeStr=single
  Product3 : Product  Inputs=4  OutDataTypeStr=single
  distPhase3 : Outport
  wires:
  tPhase1/1 -> Add/1
  tPhase2/1 -> Product1/2, Product1/1, Add/2
  subjLongAccelFilt/1 -> Product/2
  Add/1 -> Product/3
  Constant/1 -> Product/1
  Constant1/1 -> Product1/4
  Kjerk/1 -> Product1/3
  Product/1 -> Add1/2
  Product1/1 -> Add1/3
  subjVel/1 -> Add1/1
  Add1/1 -> Product2/2
  tPhase3/1 -> Product2/1, Product3/4, Product3/3
  Constant2/1 -> Product3/1
  accelMax/1 -> Product3/2
  Product3/1 -> Add2/1
  Product2/1 -> Add2/2
  Add2/1 -> distPhase3/1

== system TosAeb/FcwAebJa_Step_sys/ALG_AEBSystem/ALG_AEBController/AEB_LatObjInfoCalcApp/LatPedObjCalc/LatPedCollisonAssessInfo/LatPedCollisonAssessInfo/LatPedLongDistCondCalc/PedDistBrakeFullCalc/DistPhase3Calc/tPhase3Calc ==
  subjVel : Inport
  subjLongAccelFilt : Inport  Port=2  OutDataTypeStr=single
  tJerkWork : Inport  Port=3  OutDataTypeStr=single
  Kjerk : Inport  Port=4  OutDataTypeStr=single
  accelMax : Inport  Port=5  OutDataTypeStr=single
  velDelta : Inport  Port=6  OutDataTypeStr=single
  Action Port : ActionPort  ActionPortLabel=else
  Bus Creator : BusCreator  Inputs=6
  Bus Selector : BusSelector  OutputSignals=velDelta,subjLongAccelFilt,tJerkWork,Kjerk,accelMax
  Bus Selector1 : BusSelector  OutputSignals=subjVel,subjLongAccelFilt,tJerkWork,Kjerk,accelMax
  tEgoStopCalc : Subsystem
  tPhase3Calc : Subsystem
  tVelDeltaCalc : Subsystem
  tPhase3 : Outport
  wires:
  tVelDeltaCalc/1(tVelDelta) -> tPhase3Calc/1(tVelDelta)
  tEgoStopCalc/1(tEgoStop) -> tPhase3Calc/2(tEgoStop)
  tPhase3Calc/1(tPhase3) -> tPhase3/1
  subjVel/1 -> Bus Creator/1  "subjVel"
  subjLongAccelFilt/1 -> Bus Creator/2  "subjLongAccelFilt"
  tJerkWork/1 -> Bus Creator/3  "tJerkWork"
  Kjerk/1 -> Bus Creator/4  "Kjerk"
  accelMax/1 -> Bus Creator/5  "accelMax"
  velDelta/1 -> Bus Creator/6  "velDelta"
  Bus Creator/1 -> Bus Selector1/1, Bus Selector/1
  Bus Selector/3 -> tVelDeltaCalc/3(tJerkWork)  "<tJerkWork>"
  Bus Selector/2 -> tVelDeltaCalc/2(subjLongAccelFilt)  "<subjLongAccelFilt>"
  Bus Selector/4 -> tVelDeltaCalc/4(Kjerk)  "<Kjerk>"
  Bus Selector/1 -> tVelDeltaCalc/1(velDelta)  "<velDelta>"
  Bus Selector/5 -> tVelDeltaCalc/5(accelMax)  "<accelMax>"
  Bus Selector1/1 -> tEgoStopCalc/1(subjVel)  "<subjVel>"
  Bus Selector1/2 -> tEgoStopCalc/2(subjLongAccelFilt)  "<subjLongAccelFilt>"
  Bus Selector1/3 -> tEgoStopCalc/3(tJerkWork)  "<tJerkWork>"
  Bus Selector1/4 -> tEgoStopCalc/4(Kjerk)  "<Kjerk>"
  Bus Selector1/5 -> tEgoStopCalc/5(accelMax)  "<accelMax>"

== system TosAeb/FcwAebJa_Step_sys/ALG_AEBSystem/ALG_AEBController/AEB_LatObjInfoCalcApp/LatPedObjCalc/LatPedCollisonAssessInfo/LatPedCollisonAssessInfo/LatPedLongDistCondCalc/PedDistBrakeFullCalc/DistPhase3Calc/tPhase3Calc1 ==
  Action Port : ActionPort  ActionPortLabel=if(u1)
  Constant : Constant  Value=0  OutDataTypeStr=single
  tPhase3 : Outport
  wires:
  Constant/1 -> tPhase3/1

== system TosAeb/FcwAebJa_Step_sys/ALG_AEBSystem/ALG_AEBController/AEB_LatObjInfoCalcApp/LatPedObjCalc/LatPedObjCalc/latPedObjInfoCalc/ObjColiisionRiskProbCalc/CollisionRiskProbCalc/CollisionRiskProbCalc/CriticalityPointCalc ==
  dxReginObjLLCorr : Inport  OutDataTypeStr=single
  dyReginObjRUCorr : Inport  Port=2  OutDataTypeStr=single
  dyReginObjLLCorr : Inport  Port=3  OutDataTypeStr=single
  Add : Sum  OutDataTypeStr=single
  Gain : Gain  Gain=0.5  OutDataTypeStr=single
  dxPreCollison : Outport
  dyPreCollison : Outport  Port=2
  wires:
  dxReginObjLLCorr/1 -> dxPreCollison/1
  dyReginObjRUCorr/1 -> Add/1
  dyReginObjLLCorr/1 -> Add/2
  Add/1 -> Gain/1
  Gain/1 -> dyPreCollison/1

== system TosAeb/FcwAebJa_Step_sys/ALG_AEBSystem/ALG_AEBController/AEB_LatObjInfoCalcApp/LatPedObjCalc/LatPedObjCalc/latPedObjInfoCalc/ObjColiisionRiskProbCalc/CollisionRiskProbCalc/CollisionRiskProbCalc/pedCollisionRiskProbCalc ==
  dyOverlap : Inport  OutDataTypeStr=single
  dyOverlapLimit : Inport  Port=2  OutDataTypeStr=single
  dxOverlap : Inport  Port=3  OutDataTypeStr=single
  dxOverlapLimit : Inport  Port=4  OutDataTypeStr=single
  Constant : Constant  OutDataTypeStr=single
  Constant1 : Constant  OutDataTypeStr=single
  Constant2 : Constant  OutDataTypeStr=single
  Divide : Product  Inputs=*/  OutDataTypeStr=single
  Divide1 : Product  Inputs=*/  OutDataTypeStr=single
  MinMax : MinMax  Inputs=2  OutDataTypeStr=single
  MinMax1 : MinMax  Inputs=2  OutDataTypeStr=single
  MinMax2 : MinMax  Inputs=2  OutDataTypeStr=single
  Product : Product  OutDataTypeStr=single
  collisionRiskProb : Outport
  dyCollisionRiskProb : Outport  Port=2
  dxCollisionRiskProb : Outport  Port=3
  wires:
  MinMax/1 -> Product/1, dyCollisionRiskProb/1  "dyCollisionRiskProb"
  Constant/1 -> MinMax/2
  Divide/1 -> MinMax/1  "dyCollisionRiskProb"
  dyOverlap/1 -> Divide/1
  dyOverlapLimit/1 -> Divide/2
  MinMax1/1 -> dxCollisionRiskProb/1, Product/2  "dxCollisionRiskProb"
  Constant1/1 -> MinMax1/2
  dxOverlap/1 -> Divide1/1
  dxOverlapLimit/1 -> Divide1/2
  Divide1/1 -> MinMax1/1  "dxCollisionRiskProb"
  Product/1 -> MinMax2/1
  Constant2/1 -> MinMax2/2
  MinMax2/1 -> collisionRiskProb/1

== system TosAeb/FcwAebJa_Step_sys/ALG_AEBSystem/ALG_AEBController/AEB_LatObjInfoCalcApp/LatPedObjCalc/LatPedObjCalc/latPedObjInfoCalc/ObjColiisionRiskProbCalc/CollisionRiskProbCalc/ObjAbsInfoCalc/RadicalRelevantCalc ==
  dyTargetObjCalc : Inport
  dxTargetObjCalc : Inport  Port=2
  subjYawRate : Inport  Port=3
  Math Function : Math  Operator=hypot  SignedPower=on  SaturateOnIntegerOverflow=on
  Product1 : Product
  Trigonometric Function : Trigonometry  Operator=atan2
  radialVelRel : Outport
  objAngleToRear : Outport  Port=2
  wires:
  Trigonometric Function/1 -> objAngleToRear/1
  subjYawRate/1 -> Product1/2
  Math Function/1 -> Product1/1  "distRadial"
  Product1/1 -> radialVelRel/1  "radialVelRel"
  dyTargetObjCalc/1 -> Trigonometric Function/1, Math Function/2
  dxTargetObjCalc/1 -> Trigonometric Function/2, Math Function/1

== system TosAeb/FcwAebJa_Step_sys/ALG_AEBSystem/ALG_AEBController/AEB_LatObjInfoCalcApp/LatPedObjCalc/LatPedObjCalc/latPedObjInfoCalc/ObjColiisionRiskProbCalc/CollisionRiskProbCalc/ObjAbsInfoCalc/RadicalToXYTrans ==
  subjVel : Inport
  velRadicalXY : Inport  Port=2
  sincosCoef : Inport  Port=3
  Add3 : Sum  Inputs=++-
  Add4 : Sum
  Bus Creator : BusCreator  Inputs=2
  Bus Creator4 : BusCreator  Inputs=2
  Bus Selector10 : BusSelector  OutputSignals=velRadicalXY.velRadicalY
  Bus Selector11 : BusSelector  OutputSignals=sincosCoef.sinCoef
  Bus Selector12 : BusSelector  OutputSignals=velRadicalXY.velRadicalX
  Bus Selector13 : BusSelector  OutputSignals=sincosCoef.sinCoef
  Bus Selector14 : BusSelector  OutputSignals=velRadicalXY.velRadicalY
  Bus Selector15 : BusSelector  OutputSignals=sincosCoef.cosCoef
  Bus Selector8 : BusSelector  OutputSignals=velRadicalXY.velRadicalX
  Bus Selector9 : BusSelector  OutputSignals=sincosCoef.cosCoef
  Product{7..10} (4 alike) : Product
  velAbsObjCorr : Outport
  wires:
  Product8/1 -> Add3/3
  Bus Selector13/1 -> Product9/2  "<sinCoef>"
  Add3/1 -> Bus Creator/1  "vxAbsObjCorr"
  Product7/1 -> Add3/2
  Bus Selector14/1 -> Product10/1  "<velRadicalY>"
  Bus Selector11/1 -> Product8/2  "<sinCoef>"
  Bus Selector10/1 -> Product8/1  "<velRadicalY>"
  subjVel/1 -> Add3/1
  Add4/1 -> Bus Creator/2  "vyAbsObjCorr"
  Bus Creator4/1 -> Bus Selector15/1, Bus Selector14/1, Bus Selector13/1, Bus Selector12/1, Bus Selector11/1, Bus Selector10/1, Bus Selector9/1, Bus Selector8/1
  Bus Selector9/1 -> Product7/2  "<cosCoef>"
  Product10/1 -> Add4/2
  Bus Selector15/1 -> Product10/2  "<cosCoef>"
  Bus Selector8/1 -> Product7/1  "<velRadicalX>"
  Product9/1 -> Add4/1
  Bus Selector12/1 -> Product9/1  "<velRadicalX>"
  velRadicalXY/1 -> Bus Creator4/1
  sincosCoef/1 -> Bus Creator4/2
  Bus Creator/1 -> velAbsObjCorr/1

== system TosAeb/FcwAebJa_Step_sys/ALG_AEBSystem/ALG_AEBController/AEB_LatObjInfoCalcApp/LatPedObjCalc/LatPedObjCalc/latPedObjInfoCalc/ObjColiisionRiskProbCalc/CollisionRiskProbCalc/ObjAbsInfoCalc/XYToRadicalTrans ==
  LongLatVelRel : Inport
  radialVelRel : Inport  Port=2
  sincosCoef : Inport  Port=3
  Add : Sum
  Add2 : Sum  Inputs=+-+
  Bus Creator2 : BusCreator  Inputs=2
  Bus Creator3 : BusCreator  Inputs=2
  Bus Selector : BusSelector  OutputSignals=LongLatVelRel.vxRelTargetObjCalc
  Bus Selector1 : BusSelector  OutputSignals=sincosCoef.cosCoef
  Bus Selector2 : BusSelector  OutputSignals=LongLatVelRel.vyRelTargetObjCalc
  Bus Selector3 : BusSelector  OutputSignals=sincosCoef.sinCoef
  Bus Selector4 : BusSelector  OutputSignals=LongLatVelRel.vyRelTargetObjCalc
  Bus Selector5 : BusSelector  OutputSignals=sincosCoef.cosCoef
  Bus Selector6 : BusSelector  OutputSignals=LongLatVelRel.vxRelTargetObjCalc
  Bus Selector7 : BusSelector  OutputSignals=sincosCoef.sinCoef
  Product{2,4..6} (4 alike) : Product
  velRadicalXY : Outport
  wires:
  Product6/1 -> Add2/2
  Bus Creator3/1 -> velRadicalXY/1  "velRadicalXY"
  Product5/1 -> Add2/1
  Bus Selector2/1 -> Product4/1  "<vyRelTargetObjCalc>"
  Product4/1 -> Add/2
  Product2/1 -> Add/1
  Bus Creator2/1 -> Bus Selector7/1, Bus Selector6/1, Bus Selector5/1, Bus Selector4/1, Bus Selector3/1, Bus Selector2/1, Bus Selector1/1, Bus Selector/1
  Bus Selector5/1 -> Product5/2  "<cosCoef>"
  Add2/1 -> Bus Creator3/2  "velRadicalY"
  Bus Selector6/1 -> Product6/1  "<vxRelTargetObjCalc>"
  Add/1 -> Bus Creator3/1  "velRadicalX"
  Bus Selector4/1 -> Product5/1  "<vyRelTargetObjCalc>"
  Bus Selector1/1 -> Product2/2  "<cosCoef>"
  radialVelRel/1 -> Add2/3
  Bus Selector3/1 -> Product4/2  "<sinCoef>"
  Bus Selector/1 -> Product2/1  "<vxRelTargetObjCalc>"
  Bus Selector7/1 -> Product6/2  "<sinCoef>"
  sincosCoef/1 -> Bus Creator2/2
  LongLatVelRel/1 -> Bus Creator2/1

== system TosAeb/FcwAebJa_Step_sys/ALG_AEBSystem/ALG_AEBController/AEB_LatObjInfoCalcApp/LatPedObjCalc/LatPedObjCalc/latPedObjInfoCalc/ObjColiisionRiskProbCalc/CollisionRiskProbCalc/ObjAbsInfoCalc/sincosCoefCalc ==
  angle : Inport
  Bus Creator : BusCreator  Inputs=2
  Sincos : Trigonometry  Operator=sincos
  sincosCoef : Outport
  wires:
  Sincos/1 -> Bus Creator/1  "sinCoef"
  angle/1 -> Sincos/1
  Sincos/2 -> Bus Creator/2  "cosCoef"
  Bus Creator/1 -> sincosCoef/1  "sincosCoef"

== system TosAeb/FcwAebJa_Step_sys/ALG_AEBSystem/ALG_AEBController/AEB_LatObjInfoCalcApp/LatPedObjCalc/LatPedObjCalc/latPedObjInfoCalc/ObjColiisionRiskProbCalc/CollisionRiskProbCalc/ObjReginCorrCalc/AccelXYMaxMinCorrCalc ==
  maxAccelDecel : Inport
  velAbsTargetCorr : Inport  Port=2
  AccelXMaxMinCorrCalc : Subsystem
  AccelXYMaxMinCalc : Subsystem
  AccelYMaxMinCorrCalc : Subsystem
  Bus Creator : BusCreator  Inputs=4
  Bus Selector : BusSelector  OutputSignals=vxAbsObjCorr,vyAbsObjCorr
  Bus Selector1 : BusSelector  OutputSignals=AccelYMax,AccelYMin
  Bus Selector2 : BusSelector  OutputSignals=AccelXMax,AccelXMin
  sincosCoefCalc : Subsystem
  vXYAngleCalc : Subsystem
  AccelXMaxMinCorr : Outport
  AccelYMaxMinCorr : Outport  Port=2
  wires:
  velAbsTargetCorr/1 -> Bus Selector/1, vXYAngleCalc/1(velAbsTargetCorr)
  vXYAngleCalc/1(vXYAngle) -> sincosCoefCalc/1(angle)
  maxAccelDecel/1 -> AccelXYMaxMinCalc/1(maxAccelDecel)
  sincosCoefCalc/1(sincosCoef) -> AccelXYMaxMinCalc/2(sincosCoef)
  Bus Selector/1 -> AccelXMaxMinCorrCalc/1(vxAbsTargetCorr)  "<vxAbsObjCorr>"
  AccelXYMaxMinCalc/4(AccelYMin) -> Bus Creator/4  "AccelYMin"
  AccelXYMaxMinCalc/3(AccelYMax) -> Bus Creator/3  "AccelYMax"
  AccelXYMaxMinCalc/2(AccelXMin) -> Bus Creator/2  "AccelXMin"
  AccelXYMaxMinCalc/1(AccelXMax) -> Bus Creator/1  "AccelXMax"
  Bus Creator/1 -> Bus Selector2/1, Bus Selector1/1
  Bus Selector1/1 -> AccelYMaxMinCorrCalc/2(AccelMaxY)  "<AccelYMax>"
  Bus Selector1/2 -> AccelYMaxMinCorrCalc/3(AccelMinY)  "<AccelYMin>"
  Bus Selector2/1 -> AccelXMaxMinCorrCalc/2(AccelMaxX)  "<AccelXMax>"
  Bus Selector2/2 -> AccelXMaxMinCorrCalc/3(AccelMinX)  "<AccelXMin>"
  Bus Selector/2 -> [vyAbsTargetCorr]  "<vyAbsObjCorr>"
  [vyAbsTargetCorr] -> AccelYMaxMinCorrCalc/1(vyAbsTargetCorr)
  AccelXMaxMinCorrCalc/1(AccelXMaxMinCorr) -> AccelXMaxMinCorr/1
  AccelYMaxMinCorrCalc/1(AccelYMaxMinCorr) -> AccelYMaxMinCorr/1
